simulink model "MPC_gamecontroller"  (Simulink 7.6)
settings: Solver=ode3  StartTime=0.0  StopTime=inf  FixedStep=.05  RelTol=1e-3
InitFcn:
  | a2g = 1/9.81;
  | r2d = 180/pi;
  | K_sr = 15.97;
  | neutral_steer = 103;
  | s2t = (pi/180)*(1/K_sr);
  | A_kt =[         0         0         0         0         0         0
  |          0   -3.5058   -1.6990  -25.6114   -2.2435         0
  |          0    2.2782   -4.2575  -64.1772   -5.6218         0
  |          0         0    1.0000         0         0         0
  |          0   -0.0158    0.0295    0.4442   -4.1900         0
  |          0         0         0         0    1.0000         0];
  | 
  | 
  | B_kt =[0
  |    23.7845
  |   -16.0342
  |          0
  |    17.5770
  |          0];
  | 
  | dt = 0.05;
  | measnoise_posX = 3.36;
  | measnoise_posY = 3.95;
  | measnoise_theta = 0.136;
  | measnoise_phi =  0.078;
  | measnoise_velocity = measnoise_posX*dt;
  | 
  | accelnoiseX = 0.97;
  | accelnoiseY = 1.36;
  | gyronoisetheta = 0.04;
  | gyronoisephi = 0.021;
  | 
  | anx = accelnoiseX^2;
  | any = accelnoiseY^2;
  | anxy = accelnoiseX*accelnoiseY;
  | gntheta = gyronoisetheta^2;
  | agthetax = gyronoisetheta*accelnoiseX;
  | agthetay = gyronoisetheta*accelnoiseY;
  | gnphi = gyronoisephi^2;
  | agphix = gyronoisephi*accelnoiseX;
  | agphiy = gyronoisephi*accelnoiseY;
  | gnthetaphi = gyronoisetheta*gyronoisephi;
  | 
  | A = [1 dt 0 0 0 0;...
  |         0 1 0 0 0 0;...
  |         0 0 1 dt 0 0;...
  |         0 0 0 1 0 0;...
  |         0 0 0 0 1 0;...
  |         0 0 0 0 0 1];
  | 
  | Sw = [anx*dt^4/4, anx*dt^3/2, anxy*dt^4/4, anxy*dt^3/2,  agthetax*dt^3/2, agphix*dt^3/2;...
  |       anx*dt^3/2, anx*dt^2,   anxy*dt^3/2, anxy*dt^2, agthetax*dt^2,   agphix*dt^2;...
  |       anxy*dt^4/4, anxy*dt^3/2, any*dt^4/4, any*dt^3/2,  agthetay*dt^3/2, agphiy*dt^3/2;...
  |       anxy*dt^3/2, anxy*dt^2,   any*dt^3/2, any*dt^2, agthetay*dt^2, agphiy*dt^2;...
  |       agthetax*dt^3/2, agthetax*dt^2, agthetay*dt^3/2, agthetay*dt^2, gntheta*dt^2, gnthetaphi*dt^2;...
  |       agphix*dt^3/2, agphix*dt^2, agphiy*dt^3/2, agphiy*dt^2, gnthetaphi*dt^2, gnphi*dt^2];
  | 
  | Sz =  [measnoise_posX^2, 0, 0, 0, 0, 0;...
  |          0, measnoise_velocity^2, 0, 0, 0, 0;...
  |          0, 0, measnoise_posY^2, 0, 0, 0;...
  |          0, 0, 0, measnoise_velocity^2, 0, 0;...
  |          0, 0, 0, 0, measnoise_theta^2, 0;...
  |          0, 0, 0, 0, 0, measnoise_phi^2];
  | 
  | load MPC_test_2
StopFcn:
  | %x = x_values.signals.values;
  | %y = -y_values.signals.values;
  | %yL = y + 0.5;
  | %yR = y - 0.5;
  | 
  | %hold all;
  | 
  | %plot(x,yL,'r');
  | %plot(x,yR,'r');
  | 
  | %line([15 17],[0 0]);
  | %line([15 17],[-1 -1]);
  | %line([15 15],[0 -1]);
  | %line([17 17],[0 -1]);
  | %axis([0 30 -5 5]);
  | 
  | %hold off;
undefined symbols (not defined in the file or in its folder): CR, DiscIntNeverNeededParam, fdesignblkfcn, gcbh, serial
libraries: simulink x417, instrumentlib x3, dspadpt3 x1, realtime_pacer_lib x1, vrlib x1
(part 1 of 3)
settings Model: Creator=TCA  LastModifiedBy=ipainter  BufferReuse=off
settings OptimizationCC: BlockReduction=off  InlineParams=on  BufferReuse=off  InitFltsAndDblsToZero=on
settings DebuggingCC: AlgebraicLoopMsg=none  SolverPrmCheckMsg=none  InheritedTsInSrcMsg=none  UnconnectedInputMsg=none  UnconnectedOutputMsg=none  UnconnectedLineMsg=none  StrictBusMsg=Warning
settings SFSimCC: SimParseCustomCode=off

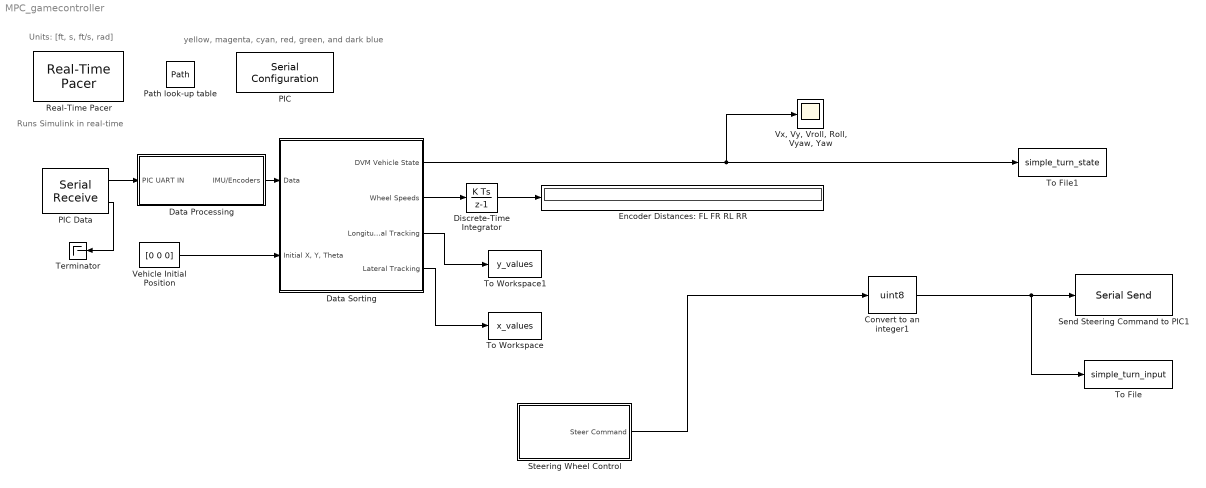
== system MPC_gamecontroller ==
  note: Runs Simulink
      |  in real-time
  note: Units: [ft, s, ft/s, rad]
  note: yellow, magenta, cyan, red, green, and dark blue
  Convert to an integer1 : DataTypeConversion  OutDataTypeStr=uint8  SampleTime=.05  SaturateOnIntegerOverflow=on  RndMeth=Zero
  Data Processing : Subsystem
  Data Sorting : Subsystem
  Discrete-Time Integrator : DiscreteIntegrator  SampleTime=0.05  ICPrevOutput=DiscIntNeverNeededParam  ICPrevScaledInput=DiscIntNeverNeededParam
  Encoder Distances: FL FR RL RR : Display
  PIC : instrumentlib/Serial Configuration  Priority=-200000  DialogController=instrumentcreatedialog  DialogControllerArgs=DataTag421  ComPortMenu=COM5  ComPort=COM5  ObjConstructor=serial('COM5');  BaudRate=9600  DataBits=8  Parity=none  StopBits=1  FlowControl=none  Timeout=10  ByteOrder=LittleEndian
  PIC Data : instrumentlib/Serial Receive  DialogController=instrumentcreatedialog  DialogControllerArgs=DataTag422  ComPortMenu=COM5  ComPort=COM5  ObjConstructor=serial('COM5');  Terminator=CR ('\r')  DataSize="[1  16]"  DataType=uint8  EnableBlockingMode=off  ActionDataUnavailable=Output last received value  CustomValue=0  SampleTime=.05
  Path look-up table : DataStoreMemory  DataStoreName=Path  ReadBeforeWriteMsg=warning  WriteAfterWriteMsg=warning  WriteAfterReadMsg=warning  InitialValue=zeros(50, 6)  VectorParams1D=off
  Real-Time Pacer : realtime_pacer_lib/Real-Time Pacer  simTimePerRealTime=1
  Send Steering Command to PIC1 : instrumentlib/Serial Send  DialogController=instrumentcreatedialog  DialogControllerArgs=DataTag423  ComPortMenu=COM5  ComPort=COM5  ObjConstructor=serial('COM5');  Header=250  Terminator=<none>  EnableBlockingMode=off
  Steering Wheel Control : Subsystem
  Terminator : Terminator
  To File : ToFile  Filename=simple_turn_input  MatrixName=ST_input  SaveFormat=Timeseries
  To File1 : ToFile  Filename=simple_turn_state  MatrixName=ST_state  SaveFormat=Timeseries
  To Workspace : ToWorkspace  VariableName=x_values  SampleTime=-1  SaveFormat=Array
  To Workspace1 : ToWorkspace  VariableName=y_values  SampleTime=-1  SaveFormat=Array
  Vehicle Initial Position : Constant  Value=[0 0 0]  VectorParams1D=off
  "Vx, Vy, Vroll, Roll, Vyaw, Yaw" : Scope
      settings: TimeRange=10  YMax=30  SaveName=ScopeData2
  wires:
  Vehicle Initial Position/1 -> Data Sorting/2("Initial X, Y, Theta")
  Data Processing/1(IMU/Encoders) -> Data Sorting/1(Data)
  Discrete-Time Integrator/1 -> Encoder Distances: FL FR RL RR/1
  Data Sorting/2(Wheel Speeds) -> Discrete-Time Integrator/1
  Data Sorting/3(Longitudinal Tracking) -> To Workspace1/1
  Data Sorting/4(Lateral Tracking) -> To Workspace/1
  Data Sorting/1(DVM Vehicle State) -> "Vx, Vy, Vroll, Roll, Vyaw, Yaw"/1, To File1/1
  Convert to an integer1/1 -> To File/1, Send Steering Command to PIC1/1
  PIC Data/2 -> Terminator/1
  PIC Data/1 -> Data Processing/1(PIC UART IN)
  Steering Wheel Control/1(Steer Command) -> Convert to an integer1/1

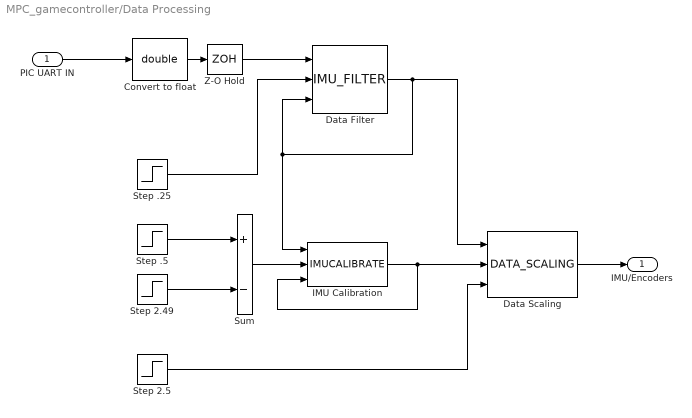
== system MPC_gamecontroller/Data Processing ==
  PIC UART IN : Inport
  Convert to float : DataTypeConversion  OutDataTypeStr=double  SaturateOnIntegerOverflow=on  RndMeth=Zero
  Data Filter : M-S-Function  FunctionName=IMU_FILTER  Parameters=3
  "Data Scaling " : M-S-Function  FunctionName=DATA_SCALING  Parameters=3
  IMU Calibration : M-S-Function  FunctionName=IMUCALIBRATE  Parameters=3
  Step .25 : Step  Time=.25  SampleTime=0
  Step .5 : Step  Time=.5  SampleTime=0
  Step 2.49 : Step  Time=2.49  SampleTime=0
  Step 2.5 : Step  Time=2.5  SampleTime=0
  Sum : Sum  Inputs=+-
  Z-O Hold : ZeroOrderHold  SampleTime=0.05
  IMU/Encoders : Outport
  wires:
  Convert to float/1 -> Z-O Hold/1
  Data Filter/1 -> "Data Scaling "/1, IMU Calibration/1, Data Filter/3
  IMU Calibration/1 -> IMU Calibration/3, "Data Scaling "/2
  Step .5/1 -> Sum/1
  Step 2.49/1 -> Sum/2
  Z-O Hold/1 -> Data Filter/1
  Step .25/1 -> Data Filter/2
  Sum/1 -> IMU Calibration/2
  Step 2.5/1 -> "Data Scaling "/3
  "Data Scaling "/1 -> IMU/Encoders/1
  PIC UART IN/1 -> Convert to float/1

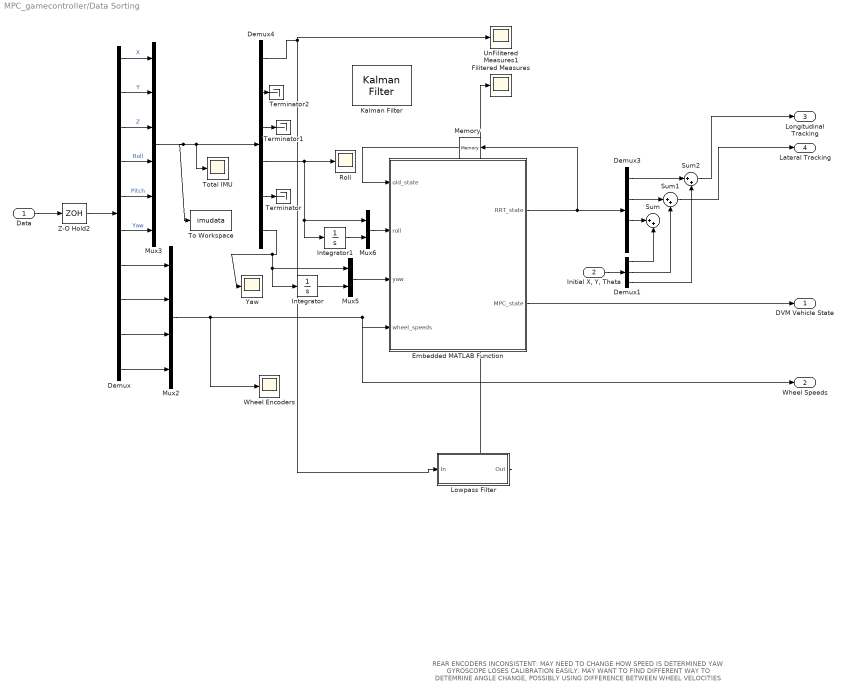
== system MPC_gamecontroller/Data Sorting ==
  note: REAR ENCODERS INCONSISTENT: MAY NEED TO CHANGE HOW SPEED IS DETERMINED
      | YAW GYROSCOPE LOSES CALIBRATION EASILY: MAY WANT TO FIND DIFFERENT WAY TO DETEMRINE
      | ANGLE CHANGE, POSSIBLY USING DIFFERENCE BETWEEN WHEEL VELOCITIES
  Data : Inport
  "Initial X, Y, Theta" : Inport  Port=2
  Demux : Demux  Outputs=10
  Demux1 : Demux  Outputs=3
  Demux3 : Demux
  Demux4 : Demux  Outputs=6
  Embedded MATLAB Function : Stateflow chart  TreatAsAtomicUnit=on
  Filitered Measures : Scope
      settings: YMin=-2.5e-034  YMax=5.25e-033  SaveName=ScopeData5
  Integrator : Integrator
  Integrator1 : Integrator
  Kalman Filter : dspadpt3/Kalman Filter  DialogController=dspDDGCreate  DialogControllerArgs=DataTag0  SystemSampleTime=-1  num_targets=1  sourceEnable=Always  isReset=on  X=0  P=10  A=0.05  sourceMeasure=Specify via Dialog  H=1  Q=10  R=10  isOutputEstMeasure=on  isOutputEstState=off  isOutputEstError=off  isOutputPrdMeasure=off  isOutputPrdState=off  isOutputPrdError=off
  Lowpass Filter : Subsystem  DialogController=fdesignblkfcn  DialogControllerArgs=DataTag2  InitFcn=fdesignblkfcn(gcbh, {'InitFcn'});
      settings: CopyFcn=fdesignblkfcn(gcbh, {'CopyFcn'});
      mask Type: Lowpass Filter
      mask Description: Design a lowpass filter
      mask Help: helpview(mapfilelocation('dspblks'), 'dsplowpassfilter')
      mask Display: disp('Lowpass')
  Memory : Memory  X0=[0,0,0,0]  InheritSampleTime=on
  Mux2 : Mux
  Mux3 : Mux  Inputs=6
  Mux5 : Mux  Inputs=2
  Mux6 : Mux  Inputs=2
  Roll : Scope
      settings: SaveName=ScopeData3
  Sum : Sum  Inputs=|++
  Sum1 : Sum  Inputs=|++
  Sum2 : Sum  Inputs=|++
  Terminator : Terminator
  Terminator1 : Terminator
  Terminator2 : Terminator
  To Workspace : ToWorkspace  VariableName=imudata  SampleTime=-1  SaveFormat=Array
  Total IMU : Scope
      settings: YMin=-7  YMax=7
  UnFilitered Measures1 : Scope
      settings: YMin=-1  YMax=1  SaveName=ScopeData6
  Wheel Encoders : Scope
  Yaw : Scope
      settings: SaveName=ScopeData4
  Z-O Hold2 : ZeroOrderHold  SampleTime=0.05
  DVM Vehicle State : Outport
  Wheel Speeds : Outport  Port=2
  Longitudinal Tracking : Outport  Port=3
  Lateral Tracking : Outport  Port=4
  wires:
  Data/1 -> Z-O Hold2/1
  "Initial X, Y, Theta"/1 -> Demux1/1
  Memory/1 -> Embedded MATLAB Function/1(old_state)
  Embedded MATLAB Function/1(RRT_state) -> Memory/1, Demux3/1
  Demux/6 -> Mux3/6  "Yaw"
  Demux/1 -> Mux3/1  "X"
  Demux/2 -> Mux3/2  "Y"
  Mux3/1 -> Demux4/1, Total IMU/1, To Workspace/1
  Demux/3 -> Mux3/3  "Z"
  Demux/4 -> Mux3/4  "Roll"
  Demux/5 -> Mux3/5  "Pitch"
  Demux/7 -> Mux2/1
  Demux/8 -> Mux2/2
  Demux/9 -> Mux2/3
  Demux/10 -> Mux2/4
  Mux2/1 -> Embedded MATLAB Function/4(wheel_speeds), Wheel Speeds/1, Wheel Encoders/1
  Z-O Hold2/1 -> Demux/1
  Demux1/1 -> Sum/2
  Demux1/2 -> Sum1/2
  Demux1/3 -> Sum2/2
  Demux3/2 -> Sum1/1
  Demux3/1 -> Sum2/1
  Demux3/3 -> Sum/1
  Sum2/1 -> Longitudinal Tracking/1
  Sum1/1 -> Lateral Tracking/1
  Demux4/4 -> Integrator1/1, Mux6/1, Roll/1
  Demux4/6 -> Integrator/1, Mux5/1, Yaw/1
  Mux5/1 -> Embedded MATLAB Function/3(yaw)
  Integrator1/1 -> Mux6/2
  Mux6/1 -> Embedded MATLAB Function/2(roll)
  Demux4/5 -> Terminator/1
  Demux4/3 -> Terminator1/1
  Embedded MATLAB Function/2(MPC_state) -> DVM Vehicle State/1
  Demux4/2 -> Terminator2/1
  Demux4/1 -> Lowpass Filter/1(In), UnFilitered Measures1/1
  Lowpass Filter/1(Out) -> Filitered Measures/1
  Integrator/1 -> Mux5/2

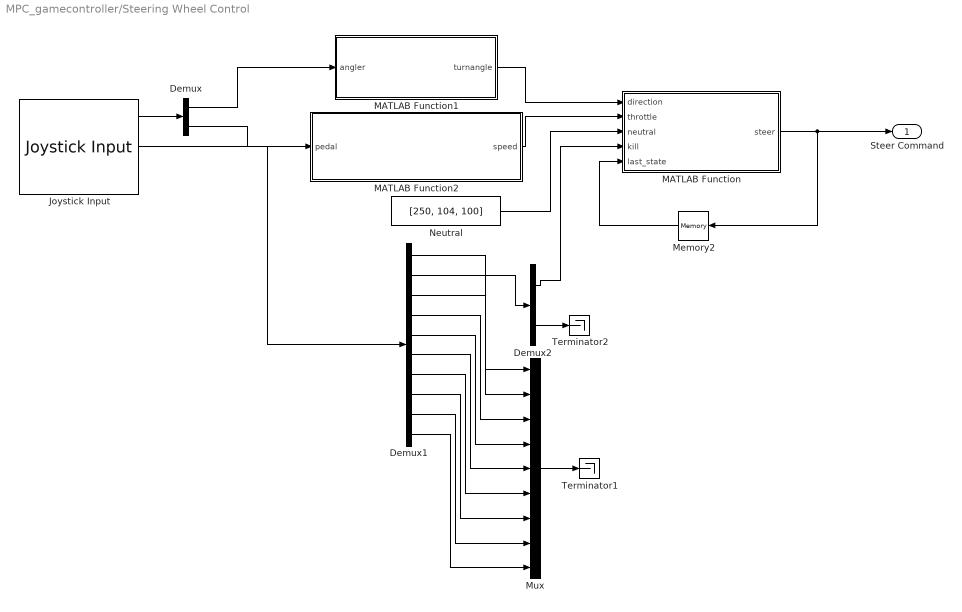
== system MPC_gamecontroller/Steering Wheel Control ==
  Demux : Demux  Outputs=2
  Demux1 : Demux  Outputs=10
  Demux2 : Demux  Outputs=2
  Joystick Input : vrlib/Joystick Input  joyid=1  adjustports=on  forcefeed=off
  MATLAB Function : Stateflow chart  TreatAsAtomicUnit=on
  MATLAB Function1 : Stateflow chart  TreatAsAtomicUnit=on
  MATLAB Function2 : Stateflow chart  TreatAsAtomicUnit=on
  Memory2 : Memory  X0=[250,104,100]  InheritSampleTime=on
  Mux : Mux  Inputs=9
  Neutral : Constant  Value=[250, 104, 100]
  Terminator1 : Terminator
  Terminator2 : Terminator
  Steer Command : Outport
  wires:
  Joystick Input/1 -> Demux/1
  MATLAB Function2/1(speed) -> MATLAB Function/2(throttle)
  Neutral/1 -> MATLAB Function/3(neutral)
  Demux1/2 -> Demux2/1
  Demux2/1 -> MATLAB Function/4(kill)
  Demux2/2 -> Terminator2/1
  Demux1/1 -> Mux/1
  Demux1/3 -> Mux/2
  Demux1/4 -> Mux/3
  Demux1/5 -> Mux/4
  Demux1/6 -> Mux/5
  Demux1/7 -> Mux/6
  Demux1/8 -> Mux/7
  Demux1/9 -> Mux/8
  Demux1/10 -> Mux/9
  Mux/1 -> Terminator1/1
  Demux/2 -> MATLAB Function2/1(pedal)
  MATLAB Function1/1(turnangle) -> MATLAB Function/1(direction)
  Demux/1 -> MATLAB Function1/1(angler)
  Joystick Input/2 -> Demux1/1
  MATLAB Function/1(steer) -> Steer Command/1, Memory2/1
  Memory2/1 -> MATLAB Function/5(last_state)

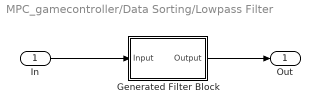
== system MPC_gamecontroller/Data Sorting/Lowpass Filter ==
  In : Inport
  Generated Filter Block : Subsystem  Tag=FilterWizardSubSystem
      settings: ErrorFcn=dspFilterRealizedInBasicElemsAlgLoopErrFcnCallback
  Out : Outport
  wires:
  In/1 -> Generated Filter Block/1(Input)
  Generated Filter Block/1(Output) -> Out/1
